simulink model "wlan_phy_rx_pmd"  (Simulink 7.8)
settings: Solver=VariableStepDiscrete  SolverMode=SingleTasking  StartTime=0.0  StopTime=simtime  RelTol=1e-3
PostLoadFcn:
  | wlan_phy_rx_init
InitFcn:
  | wlan_phy_rx_init
StartFcn:
  | wlan_phy_rx_init
values from neighbouring files (symbols this model uses):
  barker_seq20 = 0.95 * circshift(barker_seq20, [0 4]) ./ max(abs(barker_seq20))    [wlan_phy_rx_init.m]
  bit_scrambler_lfsr_bytes = bi2de(reshape(repmat(scr, 1, 8), 8, 127)', 'left-msb')    [wlan_phy_rx_init.m]
  deinterleave_ROM = [deinterleave_ROM interleave_64QAM zeros(1, 512-length(interleave_64QAM))]    [wlan_phy_rx_init.m]
  longCorr_coef_i = [3*real(LTS_corr)]    [wlan_phy_rx_init.m]
  longCorr_coef_q = [3*imag(LTS_corr)]    [wlan_phy_rx_init.m]
  MAX_CP_LEN = 32    [wlan_phy_rx_init.m]
  MAX_NCBPS = 288    [wlan_phy_rx_init.m]
  MAX_NUM_BYTES = 4096    [wlan_phy_rx_init.m]
  MAX_NUM_SC = 64    [wlan_phy_rx_init.m]
  MAX_NUM_SYMS = 600    [wlan_phy_rx_init.m]
  n_bits_preFFT_sampBuff = ceil(log2(4*MAX_NUM_SC))    [wlan_phy_rx_init.m]
  NCBPS_16QAM = 192    [wlan_phy_rx_init.m]
  NCBPS_64QAM = 288    [wlan_phy_rx_init.m]
  NCBPS_BPSK = 48    [wlan_phy_rx_init.m]
  NCBPS_QPSK = 96    [wlan_phy_rx_init.m]
  NDBPS_16QAM12 = 96    [wlan_phy_rx_init.m]
  NDBPS_16QAM34 = 144    [wlan_phy_rx_init.m]
  NDBPS_64QAM23 = 192    [wlan_phy_rx_init.m]
  NDBPS_64QAM34 = 216    [wlan_phy_rx_init.m]
  NDBPS_BPSK12 = 24    [wlan_phy_rx_init.m]
  NDBPS_BPSK34 = 36    [wlan_phy_rx_init.m]
  NDBPS_QPSK12 = 48    [wlan_phy_rx_init.m]
  NDBPS_QPSK34 = 72    [wlan_phy_rx_init.m]
  paylod_vec_samp_time = 8    [wlan_phy_rx_init.m]
  PHY_MIN_PKT_LEN = 14    [wlan_phy_rx_init.m]
  scr_ind_rev = zeros(1,128)    [wlan_phy_rx_init.m]
undefined symbols (not defined in the file or in its folder): gcbh, xlBlockLoadCallback, xlGetNormalizedPeriod, xlipgetpartsetting
libraries: xbsIndex_r4 x3119, simulink x1, xbsEDKLib_r4 x1
(part 5 of 19)

== system wlan_phy_rx_pmd/Chan Est & EQ/Chan Est ==
  note: Scale by 2^-1, since est values
      | are summed from 2 training syms
  x_re : Inport
  x_im : Inport  Port=2
  x_ind : Inport  Port=3
  x_valid : Inport  Port=4
  Sym Ind : Inport  Port=5
  Abs : Abs
  Abs1 : Abs
  Chan Est Offload : Subsystem
  Constant1 : xbsIndex_r4/Constant  const=2  gui_display_data_type=Fixed-point  arith_type=Unsigned  n_bits=2  bin_pt=0  preci_type=Single  exp_width=8  frac_width=24  explicit_period=off  period=8  equ=P=C  opselect=C  inp2=PCIN>>17  opr=+  inp1=P  carry=CIN  dbl_ovrd=off  block_type=constant
  Constant2 : xbsIndex_r4/Constant  const=0  gui_display_data_type=Fixed-point  arith_type=Unsigned  n_bits=2  bin_pt=0  preci_type=Single  exp_width=8  frac_width=24  explicit_period=off  period=8  equ=P=C  opselect=C  inp2=PCIN>>17  opr=+  inp1=P  carry=CIN  dbl_ovrd=off  block_type=constant
  Delay{1..4,7} (5 alike) : xbsIndex_r4/Delay  rst=off  infoeditControl=Selection of Reset will increase slice count due to use of real FFs and instead of SRLs  en=off  latency=2  dbl_ovrd=off  reg_retiming=off  block_type=delay
      settings: xl_area=[0, 0, 0, 0, 0, 0, 0]  block_version=VER_STRING_GOES_HERE
  Gateway Out{1..5,8} (6 alike) : xbsIndex_r4/Gateway Out  inherit_from_input=off  hdl_port=off  timing_constraint=None  locs_specified=off  LOCs={}  UseAsDAC=off  DACChannel='1'  block_type=gatewayout
  H Est Outputs : Scope  NumInputPorts=8
      settings: TimeRange=4000  YMin=-0.5~-1~-0.6000000000000001~-0.5~0~0~0~0  YMax=0.5~0.5~0.3999999999999999~1~80~1~3~0.8  SaveName=ScopeData16
  I Estimate Buffer : Subsystem
  Inverter : xbsIndex_r4/Inverter  en=off  latency=0  dbl_ovrd=off  block_type=inv
  Logical1 : xbsIndex_r4/Logical  logical_function=AND  inputs=2  en=off  latency=0  precision=Full  arith_type=Unsigned  n_bits=16  bin_pt=0  align_bp=on  dbl_ovrd=off  block_type=logical
  Logical2 : xbsIndex_r4/Logical  logical_function=AND  inputs=2  en=off  latency=0  precision=Full  arith_type=Unsigned  n_bits=16  bin_pt=0  align_bp=on  dbl_ovrd=off  block_type=logical
  Mult by LTS : Subsystem
  Q Estimate Buffer : Subsystem
  Real-Imag to Complex : RealImagToComplex
  Real-Imag to Complex1 : RealImagToComplex
  Relational1 : xbsIndex_r4/Relational  mode=a<b  en=off  latency=0  dbl_ovrd=off  block_type=relational
  Relational2 : xbsIndex_r4/Relational  mode=a=b  en=off  latency=0  dbl_ovrd=off  block_type=relational
  Scale : xbsIndex_r4/Scale  scale_factor=-1  dbl_ovrd=off  block_type=scale
  Scale1 : xbsIndex_r4/Scale  scale_factor=-1  dbl_ovrd=off  block_type=scale
  To Float : Subsystem
  " x_I" : Outport
  " x_Q" : Outport  Port=2
  H_I : Outport  Port=3
  H_Q : Outport  Port=4
  " valid" : Outport  Port=5
  " x_ind" : Outport  Port=6
  " sym_ind" : Outport  Port=7
  wires:
  Logical2/1 -> I Estimate Buffer/3(Est_WE), Q Estimate Buffer/3(Est_WE)
  Constant2/1 -> Relational2/1
  x_valid/1 -> Logical2/1, Logical1/1
  Sym Ind/1 -> Relational2/2, Relational1/1, Delay2/1
  Constant1/1 -> Relational1/2
  Relational2/1 -> I Estimate Buffer/4(First Sym), Q Estimate Buffer/4(First Sym)
  Relational1/1 -> Inverter/1, Logical2/2
  Delay7/1 -> Gateway Out4/1, To Float/5(x_ind), Chan Est Offload/1(x_ind)
  Delay1/1 -> Gateway Out5/1, To Float/6(x_valid), Chan Est Offload/4(x_valid)
  Logical1/1 -> Delay1/1
  Inverter/1 -> Logical1/2
  I Estimate Buffer/1(h[n]) -> Scale/1
  Q Estimate Buffer/1(h[n]) -> Scale1/1
  Delay2/1 -> To Float/7(sym_ind), Chan Est Offload/5(sym_ind)
  Delay3/1 -> Gateway Out8/1, To Float/1("x_I ")
  Delay4/1 -> Gateway Out1/1, To Float/2("x_Q ")
  Gateway Out8/1 -> H Est Outputs/1, Real-Imag to Complex/1  "x_I"
  Gateway Out1/1 -> H Est Outputs/2, Real-Imag to Complex/2  "x_Q"
  Gateway Out2/1 -> H Est Outputs/3, Real-Imag to Complex1/1  "H_I"
  Gateway Out3/1 -> H Est Outputs/4, Real-Imag to Complex1/2  "H_Q"
  Gateway Out4/1 -> H Est Outputs/5  "SC Ind"
  Gateway Out5/1 -> H Est Outputs/6  "Valid Out"
  x_ind/1 -> Delay7/1, Q Estimate Buffer/2(Est_Addr), I Estimate Buffer/2(Est_Addr), Mult by LTS/3(x_ind)
  x_re/1 -> Delay3/1, Mult by LTS/1(x_re)
  x_im/1 -> Delay4/1, Mult by LTS/2(x_im)
  Mult by LTS/2(H_im) -> Q Estimate Buffer/1(Est_Data)
  Mult by LTS/1(H_re) -> I Estimate Buffer/1(Est_Data)
  Scale/1 -> Gateway Out2/1, To Float/3("H_I "), Chan Est Offload/2(x_I)
  Scale1/1 -> Gateway Out3/1, To Float/4("H_Q "), Chan Est Offload/3(x_Q)
  To Float/1(" x_I") -> " x_I"/1
  To Float/2(" x_Q") -> " x_Q"/1
  To Float/3(H_I) -> H_I/1
  To Float/4(" H_Q") -> H_Q/1
  To Float/5(" x_ind") -> " x_ind"/1
  To Float/6(" x_valid") -> " valid"/1
  To Float/7(" sym_ind") -> " sym_ind"/1
  Abs/1 -> H Est Outputs/8
  Real-Imag to Complex/1 -> Abs/1
  Real-Imag to Complex1/1 -> Abs1/1
  Abs1/1 -> H Est Outputs/7

== system wlan_phy_rx_pmd/Chan Est & EQ/EQ & Pilot Processing ==
  note: Delay here so phase error is ready just in time for
      | post-EQ symbols at Phase Correction CORDIC
  x_I : Inport
  x_Q : Inport  Port=2
  H_I : Inport  Port=3
  H_Q : Inport  Port=4
  x_valid : Inport  Port=5
  x_ind : Inport  Port=6
  Sym ind : Inport  Port=7
  Convert : xbsIndex_r4/Convert  gui_display_data_type=Fixed-point  arith_type="Signed  (2's comp)"  n_bits=16  bin_pt=14  float_type=Single  exp_bits=8  fraction_bits=24  quantization="Round  (unbiased: +/- Inf)"  overflow=Saturate  en=off  latency=1  dbl_ovrd=off  pipeline=off  block_type=convert
  Convert1 : xbsIndex_r4/Convert  gui_display_data_type=Fixed-point  arith_type="Signed  (2's comp)"  n_bits=16  bin_pt=14  float_type=Single  exp_bits=8  fraction_bits=24  quantization="Round  (unbiased: +/- Inf)"  overflow=Saturate  en=off  latency=1  dbl_ovrd=off  pipeline=off  block_type=convert
  Debug Outputs : Subsystem
  Delay{1,3..5,8..9} (6 alike) : xbsIndex_r4/Delay  rst=off  infoeditControl=Selection of Reset will increase slice count due to use of real FFs and instead of SRLs  en=off  latency=62  dbl_ovrd=off  reg_retiming=off  block_type=delay
  Delay12 : xbsIndex_r4/Delay  rst=off  infoeditControl=Selection of Reset will increase slice count due to use of real FFs and instead of SRLs  en=off  latency=23  dbl_ovrd=off  reg_retiming=off  block_type=delay
  Delay14 : xbsIndex_r4/Delay  rst=off  infoeditControl=Selection of Reset will increase slice count due to use of real FFs and instead of SRLs  en=off  latency=23  dbl_ovrd=off  reg_retiming=off  block_type=delay
  Delay15 : xbsIndex_r4/Delay  rst=off  infoeditControl=Selection of Reset will increase slice count due to use of real FFs and instead of SRLs  en=off  latency=24  dbl_ovrd=off  reg_retiming=off  block_type=delay
  Delay17 : xbsIndex_r4/Delay  rst=off  infoeditControl=Selection of Reset will increase slice count due to use of real FFs and instead of SRLs  en=off  latency=23  dbl_ovrd=off  reg_retiming=off  block_type=delay
  Delay18 : xbsIndex_r4/Delay  rst=off  infoeditControl=Selection of Reset will increase slice count due to use of real FFs and instead of SRLs  en=off  latency=24  dbl_ovrd=off  reg_retiming=off  block_type=delay
  Delay2 : xbsIndex_r4/Delay  rst=off  infoeditControl=Selection of Reset will increase slice count due to use of real FFs and instead of SRLs  en=off  latency=8  dbl_ovrd=off  reg_retiming=off  block_type=delay
  Delay6 : xbsIndex_r4/Delay  rst=off  infoeditControl=Selection of Reset will increase slice count due to use of real FFs and instead of SRLs  en=off  latency=8  dbl_ovrd=off  reg_retiming=off  block_type=delay
  Delay7 : xbsIndex_r4/Delay  rst=off  infoeditControl=Selection of Reset will increase slice count due to use of real FFs and instead of SRLs  en=off  latency=8  dbl_ovrd=off  reg_retiming=off  block_type=delay
  Divide : xbsIndex_r4/Divide  flow_control=NonBlocking  axi_optimize_goal=Resources  algorithm_type=High_Radix  infoedit1=Specify output fraction-width for Fixed-point division.  fractional_width=16  latency=21  rate=1  has_a_tlast=off  has_a_tuser=off  has_b_tlast=off  has_b_tuser=off  en=off  has_result_tready=off  result_tlast_behv=Pass_A_TLAST  has_underflow=off  has_overflow=off  has_invalid_op=off  has_divide_by_zero=off  fpo_op_type=mergeddivide  block_type=divide
      settings: infoedit=Blocking: Wait for data on all inputs, allow back-pressure.<br>NonBlocking: operate every cycle in which all inputs are valid, no back-pressure.
  Divide1 : xbsIndex_r4/Divide  flow_control=NonBlocking  axi_optimize_goal=Resources  algorithm_type=High_Radix  infoedit1=Specify output fraction-width for Fixed-point division.  fractional_width=16  latency=21  rate=1  has_a_tlast=off  has_a_tuser=off  has_b_tlast=off  has_b_tuser=off  en=off  has_result_tready=off  result_tlast_behv=Pass_A_TLAST  has_underflow=off  has_overflow=off  has_invalid_op=off  has_divide_by_zero=off  fpo_op_type=mergeddivide  block_type=divide
      settings: infoedit=Blocking: Wait for data on all inputs, allow back-pressure.<br>NonBlocking: operate every cycle in which all inputs are valid, no back-pressure.
  EQ : Scope  NumInputPorts=8
      settings: TimeRange=6000  YMin=-1~-0.5~0~0~0~-2~-1~0  YMax=1~1~1~100~3~2~2~1  SaveName=ScopeData9
  Gateway Out{,1..7} (8 alike) : xbsIndex_r4/Gateway Out  inherit_from_input=off  hdl_port=off  timing_constraint=None  locs_specified=off  LOCs={}  UseAsDAC=off  DACChannel='1'  block_type=gatewayout
  Mag : Subsystem
  Phase Correction : Subsystem
  Phase Err Calc : Subsystem
  Register3 : xbsIndex_r4/Register  init=0  rst=off  en=off  dbl_ovrd=off  block_type=register
  Register4 : xbsIndex_r4/Register  init=0  rst=off  en=off  dbl_ovrd=off  block_type=register
  Register5 : xbsIndex_r4/Register  init=0  rst=off  en=off  dbl_ovrd=off  block_type=register
  a*conj(b) : Subsystem
  " x_I" : Outport
  " x_Q" : Outport  Port=2
  " x_ind" : Outport  Port=3
  " x_valid" : Outport  Port=4
  " sym ind" : Outport  Port=5
  wires:
  x_ind/1 -> Gateway Out5/1, Delay2/1
  Sym ind/1 -> Gateway Out6/1, Delay7/1
  x_I/1 -> a*conj(b)/1(A_I), Gateway Out/1
  x_Q/1 -> a*conj(b)/2(A_Q), Gateway Out1/1
  H_I/1 -> a*conj(b)/3(B_I), Mag/1(I)
  H_Q/1 -> a*conj(b)/4(B_Q), Mag/2(Q)
  x_valid/1 -> Gateway Out4/1, Delay6/1
  Delay1/1 -> Register4/1
  Delay3/1 -> Register3/1
  Phase Err Calc/1(Sym Phase Err) -> Phase Correction/3(Phase)
  Delay7/1 -> Phase Err Calc/5(sym_ind), Delay9/1
  a*conj(b)/1(P_I) -> Phase Err Calc/1(CP_I), Delay1/1
  a*conj(b)/2(P_Q) -> Phase Err Calc/2(CP_Q), Delay3/1
  Delay2/1 -> Phase Err Calc/4(x_ind), Delay5/1
  Mag/1(M) -> Delay8/1
  Delay8/1 -> Register5/1
  Divide/1 -> Convert/1
  Divide1/1 -> Convert1/1
  Convert/1 -> Phase Correction/1(x_I)
  Convert1/1 -> Phase Correction/2(x_Q)
  Register3/1 -> Divide1/1
  Register4/1 -> Divide/1
  Delay4/1 -> Delay12/1
  Delay12/1 -> Phase Correction/4(x_valid)
  Phase Correction/1(" x_I") -> " x_I"/1, Gateway Out7/1, Debug Outputs/1(I)
  Phase Correction/2(" x_Q") -> " x_Q"/1, Gateway Out2/1, Debug Outputs/2(Q)
  Phase Correction/3(" x_valid") -> " x_valid"/1, Gateway Out3/1, Debug Outputs/3(valid)
  Delay5/1 -> Delay14/1
  Delay14/1 -> Delay15/1
  Delay15/1 -> " x_ind"/1, Debug Outputs/4(x_ind)
  Delay9/1 -> Delay17/1
  Delay17/1 -> Delay18/1
  Delay18/1 -> " sym ind"/1
  Delay6/1 -> Phase Err Calc/3(x_valid), Delay4/1
  Gateway Out6/1 -> EQ/5  "Sym Ind"
  Gateway Out5/1 -> EQ/4  "X Ind"
  Gateway Out4/1 -> EQ/3  "X Valid"
  Gateway Out2/1 -> EQ/7  "EQ Q"
  Gateway Out1/1 -> EQ/2  "X_Q"
  Gateway Out/1 -> EQ/1  "X_I"
  Gateway Out7/1 -> EQ/6  "EQ I"
  Gateway Out3/1 -> EQ/8  "EQ Valid"
  Register5/1 -> Divide/2, Divide1/2

== system wlan_phy_rx_pmd/DSSS Rx/Demod ==
  Start : Inport
  I : Inport  Port=2
  Q : Inport  Port=3
  Bit-to-Byte : Subsystem
  DBPSK Demod : Subsystem
  DSSS Rx : Scope  NumInputPorts=7
      settings: TimeRange=80000  YMin=-0.75~-0.3~0~0.95~40~0~0  YMax=0.75~0.1~1~1.05~48~1~250
  De-spread : Subsystem
  Descramble : Subsystem
  Gateway Out{1..7} (7 alike) : xbsIndex_r4/Gateway Out  inherit_from_input=off  hdl_port=off  timing_constraint=None  locs_specified=off  LOCs={}  UseAsDAC=off  DACChannel='1'  block_type=gatewayout
  Header CRC : Subsystem
  Register8 : xbsIndex_r4/Register  init=0  rst=off  en=on  dbl_ovrd=off  block_type=register
  Sync : Subsystem
  Byte : Outport
  Byte Valid : Outport  Port=2
  Byte Ind : Outport  Port=3
  CRC Calc : Outport  Port=4
  wires:
  Q/1 -> De-spread/2(Q), Gateway Out3/1
  Start/1 -> De-spread/3(Sym Start), Gateway Out6/1
  I/1 -> De-spread/1(I), Gateway Out2/1
  De-spread/1(IQ Valid) -> DBPSK Demod/1(IQ Valid)
  De-spread/2(" I") -> DBPSK Demod/2(I)
  De-spread/3(" Q") -> DBPSK Demod/3(Q)
  DBPSK Demod/1(bit) -> Descramble/1(Bit)
  DBPSK Demod/2(bit valid) -> Descramble/2(Bit Valid)
  Descramble/1(" Bit") -> Register8/1, Sync/1(Bit)
  Descramble/2(" Bit Valid") -> Register8/2, Sync/2(Bit Valid)
  Gateway Out2/1 -> DSSS Rx/1
  Gateway Out3/1 -> DSSS Rx/2
  Gateway Out6/1 -> DSSS Rx/3
  Gateway Out7/1 -> DSSS Rx/4
  Gateway Out1/1 -> DSSS Rx/5
  Gateway Out4/1 -> DSSS Rx/6
  Gateway Out5/1 -> DSSS Rx/7
  Sync/1(Payload Bit) -> Bit-to-Byte/1(Bit), Header CRC/1(Bit)
  Sync/2(" Bit Valid") -> Bit-to-Byte/2(Bit Valid), Header CRC/2(Bit Valid)
  Bit-to-Byte/3(Byte Ind) -> Byte Ind/1, Gateway Out1/1
  Bit-to-Byte/1(Byte) -> Byte/1, Gateway Out5/1
  Bit-to-Byte/2(Byte Valid) -> Byte Valid/1, Gateway Out4/1
  Register8/1 -> Gateway Out7/1
  Header CRC/1(CRC Calc) -> CRC Calc/1

== system wlan_phy_rx_pmd/DSSS Rx/Reset Gen ==
  note: Extend the DSSS reset event long enough to flush
      | the DSSS PHY pipeline and to reduce the
      | chance of spurious double-detections
  Reset : Inport
  Constant1 : xbsIndex_r4/Constant  const=127  gui_display_data_type=Fixed-point  arith_type=Unsigned  n_bits=7  bin_pt=0  preci_type=Single  exp_width=8  frac_width=24  explicit_period=off  period=1  equ=P=C  opselect=C  inp2=PCIN>>17  opr=+  inp1=P  carry=CIN  dbl_ovrd=off  block_type=constant
      settings: block_version=VER_STRING_GOES_HERE
  Counter1 : xbsIndex_r4/Counter  cnt_type=Free Running  cnt_to=Inf  operation=Up  start_count=0  cnt_by_val=1  arith_type=Unsigned  n_bits=7  bin_pt=0  load_pin=off  rst=on  en=on  explicit_period=off  period=1  dbl_ovrd=off  use_behavioral_HDL=off  implementation=Fabric  block_type=counter
  Down Sample3 : xbsIndex_r4/Down Sample  sample_ratio=8  sample_phase="Last Value of Frame  (most efficient)"  en=off  latency=1  dbl_ovrd=off  block_type=dsamp
  Inverter2 : xbsIndex_r4/Inverter  en=off  latency=0  dbl_ovrd=off  block_type=inv
  Logical1 : xbsIndex_r4/Logical  logical_function=OR  inputs=2  en=off  latency=0  precision=Full  arith_type=Unsigned  n_bits=8  bin_pt=2  align_bp=on  dbl_ovrd=off  block_type=logical
      settings: xl_area=[1 0 0 1 0 0 0]  block_version=VER_STRING_GOES_HERE
  Posedge : Subsystem
  Relational1 : xbsIndex_r4/Relational  mode=a=b  en=off  latency=0  dbl_ovrd=off  block_type=relational
  S-R Latch : Subsystem
  S-R Latch2 : Subsystem
  negedge : Subsystem
  DSSS Rx Reset : Outport
  wires:
  Posedge/1(Q) -> S-R Latch/1(S), Logical1/1
  S-R Latch/1(Q) -> Down Sample3/1
  Counter1/1 -> Relational1/1
  Constant1/1 -> Relational1/2
  negedge/1(Q) -> S-R Latch2/1(S)
  S-R Latch2/1(Q) -> Counter1/2, Inverter2/1
  Relational1/1 -> S-R Latch2/2(R), S-R Latch/2(R)
  Inverter2/1 -> Logical1/2
  Reset/1 -> Posedge/1(D), negedge/1(D)
  Down Sample3/1 -> DSSS Rx Reset/1
  Logical1/1 -> Counter1/1

== system wlan_phy_rx_pmd/DSSS Rx/S-R Latch1 ==
  S : Inport
  R : Inport  Port=2
  Constant1 : xbsIndex_r4/Constant  const=1  gui_display_data_type=Boolean  arith_type=Boolean  n_bits=1  bin_pt=0  preci_type=Single  exp_width=8  frac_width=24  explicit_period=off  period=1  equ=P=C  opselect=C  inp2=PCIN>>17  opr=+  inp1=P  carry=CIN  dbl_ovrd=off  block_type=constant
      settings: block_version=VER_STRING_GOES_HERE
  Convert1 : xbsIndex_r4/Convert  gui_display_data_type=Boolean  arith_type=Boolean  n_bits=1  bin_pt=0  float_type=Single  exp_bits=8  fraction_bits=24  quantization=Truncate  overflow=Wrap  en=off  latency=0  dbl_ovrd=off  pipeline=off  block_type=convert
  Convert2 : xbsIndex_r4/Convert  gui_display_data_type=Boolean  arith_type=Boolean  n_bits=1  bin_pt=0  float_type=Single  exp_bits=8  fraction_bits=24  quantization=Truncate  overflow=Wrap  en=off  latency=0  dbl_ovrd=off  pipeline=off  block_type=convert
  Register2 : xbsIndex_r4/Register  init=0  rst=on  en=on  dbl_ovrd=off  block_type=register
      settings: xl_area=[1 1 0 0 0 0 0]  block_version=VER_STRING_GOES_HERE
  Q : Outport
  wires:
  S/1 -> Convert2/1
  Register2/1 -> Q/1
  R/1 -> Convert1/1
  Constant1/1 -> Register2/1
  Convert1/1 -> Register2/2
  Convert2/1 -> Register2/3

== system wlan_phy_rx_pmd/DSSS Rx/SIGNAL Dec ==
  note: Convert to bytes, pad for FCS
  Byte : Inport
  Byte Valid : Inport  Port=2
  Byte Ind : Inport  Port=3
  CRC Calc : Inport  Port=4
  AddSub : xbsIndex_r4/AddSub  mode=Addition  use_carryin=off  use_carryout=off  en=off  latency=0  precision=User Defined  arith_type=Unsigned  n_bits=ceil(log2(MAX_NUM_BYTES))  bin_pt=0  quantization=Truncate  overflow=Wrap  dbl_ovrd=off  use_behavioral_HDL=off  hw_selection=Fabric  pipelined=off  block_type=addsub
  Concat : xbsIndex_r4/Concat  num_inputs=2  dbl_ovrd=off  block_type=concat
  Concat1 : xbsIndex_r4/Concat  num_inputs=2  dbl_ovrd=off  block_type=concat
  Constant1 : xbsIndex_r4/Constant  const=2  gui_display_data_type=Fixed-point  arith_type=Unsigned  n_bits=2  bin_pt=0  preci_type=Single  exp_width=8  frac_width=24  explicit_period=off  period=1  equ=P=C  opselect=C  inp2=PCIN>>17  opr=+  inp1=P  carry=CIN  dbl_ovrd=off  block_type=constant
  Constant2 : xbsIndex_r4/Constant  const=0  gui_display_data_type=Fixed-point  arith_type=Unsigned  n_bits=2  bin_pt=0  preci_type=Single  exp_width=8  frac_width=24  explicit_period=off  period=1  equ=P=C  opselect=C  inp2=PCIN>>17  opr=+  inp1=P  carry=CIN  dbl_ovrd=off  block_type=constant
  Constant3 : xbsIndex_r4/Constant  const=10  gui_display_data_type=Fixed-point  arith_type=Unsigned  n_bits=8  bin_pt=0  preci_type=Single  exp_width=8  frac_width=24  explicit_period=off  period=1  equ=P=C  opselect=C  inp2=PCIN>>17  opr=+  inp1=P  carry=CIN  dbl_ovrd=off  block_type=constant
  Constant4 : xbsIndex_r4/Constant  const=3  gui_display_data_type=Fixed-point  arith_type=Unsigned  n_bits=2  bin_pt=0  preci_type=Single  exp_width=8  frac_width=24  explicit_period=off  period=1  equ=P=C  opselect=C  inp2=PCIN>>17  opr=+  inp1=P  carry=CIN  dbl_ovrd=off  block_type=constant
  Constant5 : xbsIndex_r4/Constant  const=8*1500  gui_display_data_type=Fixed-point  arith_type=Unsigned  n_bits=14  bin_pt=0  preci_type=Single  exp_width=8  frac_width=24  explicit_period=off  period=1  equ=P=C  opselect=C  inp2=PCIN>>17  opr=+  inp1=P  carry=CIN  dbl_ovrd=off  block_type=constant
  Constant6 : xbsIndex_r4/Constant  const=4  gui_display_data_type=Fixed-point  arith_type=Unsigned  n_bits=3  bin_pt=0  preci_type=Single  exp_width=8  frac_width=24  explicit_period=off  period=1  equ=P=C  opselect=C  inp2=PCIN>>17  opr=+  inp1=P  carry=CIN  dbl_ovrd=off  block_type=constant
  Constant7 : xbsIndex_r4/Constant  const=5  gui_display_data_type=Fixed-point  arith_type=Unsigned  n_bits=3  bin_pt=0  preci_type=Single  exp_width=8  frac_width=24  explicit_period=off  period=1  equ=P=C  opselect=C  inp2=PCIN>>17  opr=+  inp1=P  carry=CIN  dbl_ovrd=off  block_type=constant
  Constant8 : xbsIndex_r4/Constant  const=82  gui_display_data_type=Fixed-point  arith_type=Unsigned  n_bits=16  bin_pt=0  preci_type=Single  exp_width=8  frac_width=24  explicit_period=on  period=8  equ=P=C  opselect=C  inp2=PCIN>>17  opr=+  inp1=P  carry=CIN  dbl_ovrd=off  block_type=constant
  DOUT{,2,4..5} (4 alike) : xbsIndex_r4/Register  init=0  rst=on  en=on  dbl_ovrd=off  block_type=register
  DOUT3 : xbsIndex_r4/Register  init=4  rst=on  en=on  dbl_ovrd=off  block_type=register
  Delay1 : xbsIndex_r4/Delay  rst=off  infoeditControl=Selection of Reset will increase slice count due to use of real FFs and instead of SRLs  en=off  latency=1  dbl_ovrd=off  reg_retiming=off  block_type=delay
  Display : Display
  Display1 : Display
  Down Sample2 : xbsIndex_r4/Down Sample  sample_ratio=8  sample_phase="Last Value of Frame  (most efficient)"  en=off  latency=1  dbl_ovrd=off  block_type=dsamp
  Gateway Out : xbsIndex_r4/Gateway Out  inherit_from_input=off  hdl_port=off  timing_constraint=None  locs_specified=off  LOCs={}  UseAsDAC=off  DACChannel='1'  block_type=gatewayout
  Gateway Out1 : xbsIndex_r4/Gateway Out  inherit_from_input=off  hdl_port=off  timing_constraint=None  locs_specified=off  LOCs={}  UseAsDAC=off  DACChannel='1'  block_type=gatewayout
  Inverter1 : xbsIndex_r4/Inverter  en=off  latency=0  dbl_ovrd=off  block_type=inv
  Logical{,1..3,7..10} (8 alike) : xbsIndex_r4/Logical  logical_function=AND  inputs=2  en=off  latency=0  precision=Full  arith_type=Unsigned  n_bits=16  bin_pt=0  align_bp=on  dbl_ovrd=off  block_type=logical
  Logical6 : xbsIndex_r4/Logical  logical_function=OR  inputs=3  en=off  latency=0  precision=Full  arith_type=Unsigned  n_bits=16  bin_pt=0  align_bp=on  dbl_ovrd=off  block_type=logical
  Relational{1..2,4,6..7} (5 alike) : xbsIndex_r4/Relational  mode=a=b  en=off  latency=0  dbl_ovrd=off  block_type=relational
  Relational3 : xbsIndex_r4/Relational  mode=a!=b  en=off  latency=0  dbl_ovrd=off  block_type=relational
  Relational5 : xbsIndex_r4/Relational  mode=a>b  en=off  latency=0  dbl_ovrd=off  block_type=relational
  Relational8 : xbsIndex_r4/Relational  mode=a!=b  en=off  latency=0  dbl_ovrd=off  block_type=relational
  Shift : xbsIndex_r4/Shift  shift_dir=Right  shift_bits=3  en=off  latency=0  precision=Full  arith_type="Signed  (2's comp)"  n_bits=16  bin_pt=14  quantization=Truncate  overflow=Wrap  dbl_ovrd=off  block_type=shift
  LENGTH : Outport
  Valid Header : Outport  Port=2
  Error : Outport  Port=3
  wires:
  Constant2/1 -> Relational2/2
  Relational1/1 -> Logical/2
  Relational2/1 -> Logical2/2
  Logical/1 -> DOUT2/3
  Logical2/1 -> DOUT/3
  Byte/1 -> DOUT/1, DOUT2/1, DOUT3/1, DOUT4/1, DOUT5/1
  [DSSS_RX_RESET] -> DOUT/2, DOUT2/2, DOUT3/2, DOUT4/2, DOUT5/2
  Byte Valid/1 -> Logical2/1, Logical/1, Logical3/1, Logical8/1, Logical7/1
  Constant1/1 -> Relational1/2
  Byte Ind/1 -> Relational1/1, Relational4/1, Relational7/1, Relational6/1, Relational2/1
  DOUT/1 -> Relational3/1, Gateway Out1/1
  Constant3/1 -> Relational3/2
  Relational4/1 -> Logical3/2
  Constant4/1 -> Relational4/2
  Logical3/1 -> DOUT3/3
  DOUT2/1 -> Concat/2
  DOUT3/1 -> Concat/1
  Concat/1 -> Gateway Out/1, Relational5/1, Shift/1
  Constant5/1 -> Relational5/2
  Relational5/1 -> Logical6/2
  Relational3/1 -> Logical6/1
  Gateway Out/1 -> Display/1
  Gateway Out1/1 -> Display1/1
  Constant6/1 -> Relational6/2
  Logical8/1 -> DOUT4/3, Delay1/1
  Relational6/1 -> Logical7/2
  Relational7/1 -> Logical8/2
  Constant7/1 -> Relational7/2
  DOUT4/1 -> Concat1/1
  DOUT5/1 -> Concat1/2
  Concat1/1 -> Relational8/1
  CRC Calc/1 -> Relational8/2
  Relational8/1 -> Logical9/1
  Delay1/1 -> Logical9/2, Logical10/2, Logical1/2
  Logical9/1 -> Logical6/3
  Logical7/1 -> DOUT5/3
  [regRx_DSSS_LENGTH_PAD] -> Down Sample2/1
  Down Sample2/1 -> AddSub/2
  AddSub/1 -> LENGTH/1
  Logical6/1 -> Inverter1/1, Logical1/1
  Inverter1/1 -> Logical10/1
  Logical10/1 -> Valid Header/1
  Logical1/1 -> Error/1
  Shift/1 -> AddSub/1

== system wlan_phy_rx_pmd/DSSS Rx/Sym Sync ==
  En : Inport
  Rx I : Inport  Port=2
  Rx Q : Inport  Port=3
  ASR : xbsIndex_r4/Addressable Shift Register  infer_latency=on  depth=2  initVector=[0]  en=off  dbl_ovrd=off  use_behavioral_HDL=off  Optimization=Resource  block_type=addrsr
      settings: infoedit="Delay of configurable length.  Any element in the delay line can be addressed and driven onto the output port.<br><br>Hardware notes: This block is implemented using SRL16s. If Virtex-4 or Spartan-3 device is used, multiple SRLC16s are cascaded together."
  Abs : Subsystem
  Convert : xbsIndex_r4/Convert  gui_display_data_type=Fixed-point  arith_type="Signed  (2's comp)"  n_bits=20  bin_pt=14  float_type=Single  exp_bits=8  fraction_bits=24  quantization=Truncate  overflow=Wrap  en=off  latency=0  dbl_ovrd=off  pipeline=off  block_type=convert
  DSSS Corr : Scope  NumInputPorts=7
      settings: TimeRange=80000  YMin=-0.25~-1.5~0~0~0~0~0  YMax=0.15~4.5~20~1~1~1~1
  Delay : xbsIndex_r4/Delay  rst=off  infoeditControl=Selection of Reset will increase slice count due to use of real FFs and instead of SRLs  en=off  latency=20  dbl_ovrd=off  reg_retiming=off  block_type=delay
  Delay1 : xbsIndex_r4/Delay  rst=off  infoeditControl=Selection of Reset will increase slice count due to use of real FFs and instead of SRLs  en=off  latency=20  dbl_ovrd=off  reg_retiming=off  block_type=delay
  Display : Display
  Down Sample1 : xbsIndex_r4/Down Sample  sample_ratio=8  sample_phase="Last Value of Frame  (most efficient)"  en=off  latency=1  dbl_ovrd=off  block_type=dsamp
  Down Sample2 : xbsIndex_r4/Down Sample  sample_ratio=8  sample_phase="Last Value of Frame  (most efficient)"  en=off  latency=1  dbl_ovrd=off  block_type=dsamp
  "FIR Compiler 6.3 " : xbsIndex_r4/FIR Compiler 6.3   coefficientvector=fliplr(barker_seq20)  coefficient_sets=1  coefficient_reload=off  filter_type=Single_Rate  rate_change_type=Integer  interpolation_rate=1  decimation_rate=1  zero_pack_factor=1  channel_sequence=Basic  number_channels=1  pattern_list='P4-0,P4-1,P4-2,P4-3,P4-4'  number_paths=2  ratespecification=Maximum_Possible  sampleperiod=8  hardwareoversamplingrate=1  coefficient_sign=Signed  quantization=Maximize_Dynamic_Range  coefficient_width=16  bestprecision=on  coefficient_fractional_bits=15  coefficient_structure=Inferred  output_rounding_mode=Truncate_LSBs  output_width=16  filter_architecture=Systolic_Multiply_Accumulate  optimization_goal=Area  data_buffer_type=Automatic  coefficient_buffer_type=Automatic  input_buffer_type=Automatic  output_buffer_type=Automatic  preference_for_other_storage=Automatic  multi_column_support=Automatic  columnconfig='20'  inter_column_pipe_length=4  data_has_tlast=Not_Required  m_data_has_tready=off  s_data_has_fifo=off  s_data_has_tuser=Not_Required  m_data_has_tuser=Not_Required  s_config_sync_mode=On_Vector  s_config_method=Single  num_reload_slots=1  has_aclken=off  has_aresetn=off  infoeditControl=ARESETn must be asserted for a minimum of 2 cycles  trim_axipin_name=on  passband_min=0.0  passband_max=0.5  stopband_min=0.5  stopband_max=1.0  filter_selection=1  gui_behaviour=Sysgen_GUI  data_sign=Signed  data_width=16  data_fractional_bits=0  data_tuser_width=1  wrapper_available=true  ip_wrap_arbitrary_binary_point=true  ip_name=FIR Compiler  ip_version=6.3  dsptool_ready=true  ipcore_usecache=true  ipcore_useipmodelcache=true  ipcore_xco_need_fpga_part=true  port_translation_map={ 'aclken' => 'en', 'aresetn' => 'rst' }  run_core_at_system_period=true  ipcore_fpga_part=xlipgetpartsetting(gcb, {'virtex6', 'xc6vlx75t', '-3', 'ff784'})  block_type=fir_compiler_v6_3
      settings: infoedit=Maximum Possible : Maximizes oversampling rate. Abstracted input handshake (no s_data_tvalid) and automatic rate propagation.<br>Hardware Oversampling Rate : Specifies oversampling rate relative to the input sample period. Abstracted input handshake (no s_data_tvalid) and automatic rate propagation.<br>Sample Period : Specifies absolute oversampling rate. No input handshake abstraction (exposes s_data_tvalid) and no rate propagation.
  Gateway Out{,1..7} (8 alike) : xbsIndex_r4/Gateway Out  inherit_from_input=off  hdl_port=off  timing_constraint=None  locs_specified=off  LOCs={}  UseAsDAC=off  DACChannel='1'  block_type=gatewayout
  Logical : xbsIndex_r4/Logical  logical_function=AND  inputs=4  en=off  latency=0  precision=Full  arith_type=Unsigned  n_bits=16  bin_pt=0  align_bp=on  dbl_ovrd=off  block_type=logical
  Relational : xbsIndex_r4/Relational  mode=a<b  en=off  latency=0  dbl_ovrd=off  block_type=relational
  Sym : Outport
  tag scope: CS_DSSS_Corr=global
  wires:
  Rx Q/1 -> Gateway Out/1, "FIR Compiler 6.3 "/2
  Gateway Out/1 -> DSSS Corr/1
  "FIR Compiler 6.3 "/2 -> Abs/1(Valid)
  "FIR Compiler 6.3 "/3 -> Abs/2(I), Gateway Out1/1
  "FIR Compiler 6.3 "/4 -> Abs/3(Q)
  Gateway Out1/1 -> DSSS Corr/2
  Abs/1(A) -> Relational/2, Gateway Out2/1, Convert/1
  [regRx_DSSS_RX_CODE_CORR_THRESH] -> Down Sample2/1
  Rx I/1 -> "FIR Compiler 6.3 "/1
  Relational/1 -> ASR/1
  Gateway Out2/1 -> DSSS Corr/3
  Gateway Out3/1 -> Display/1
  ASR/1 -> Logical/1, Delay/1, Gateway Out4/1
  [regRx_DSSS_RX_DESPREAD_DLY] -> Down Sample1/1
  Delay/1 -> Delay1/1, Logical/2, Gateway Out5/1
  Delay1/1 -> Logical/3, Gateway Out6/1
  En/1 -> Logical/4
  Gateway Out4/1 -> DSSS Corr/4
  Gateway Out5/1 -> DSSS Corr/5
  Gateway Out6/1 -> DSSS Corr/6
  Gateway Out7/1 -> DSSS Corr/7
  Logical/1 -> Sym/1, Gateway Out7/1
  Down Sample1/1 -> ASR/2
  Down Sample2/1 -> Relational/1, Gateway Out3/1
  Convert/1 -> [CS_DSSS_Corr]

== system wlan_phy_rx_pmd/Detect & Decode/Ctrl ==
  Reset : Inport
  OFDM Sym Ind : Inport  Port=2
  Constant1 : xbsIndex_r4/Constant  const=1  gui_display_data_type=Fixed-point  arith_type=Unsigned  n_bits=2  bin_pt=0  preci_type=Single  exp_width=8  frac_width=24  explicit_period=off  period=1  equ=P=C  opselect=C  inp2=PCIN>>17  opr=+  inp1=P  carry=CIN  dbl_ovrd=off  block_type=constant
  Constant3 : xbsIndex_r4/Constant  const=2  gui_display_data_type=Fixed-point  arith_type=Unsigned  n_bits=2  bin_pt=0  preci_type=Single  exp_width=8  frac_width=24  explicit_period=off  period=1  equ=P=C  opselect=C  inp2=PCIN>>17  opr=+  inp1=P  carry=CIN  dbl_ovrd=off  block_type=constant
  Logical1 : xbsIndex_r4/Logical  logical_function=OR  inputs=2  en=off  latency=0  precision=Full  arith_type=Unsigned  n_bits=8  bin_pt=2  align_bp=on  dbl_ovrd=off  block_type=logical
      settings: xl_area=[1 0 0 1 0 0 0]  block_version=VER_STRING_GOES_HERE
  Posedge : Subsystem
  Posedge1 : Subsystem
  Relational1 : xbsIndex_r4/Relational  mode=a>b  en=off  latency=0  dbl_ovrd=off  block_type=relational
  Relational2 : xbsIndex_r4/Relational  mode=a>b  en=off  latency=0  dbl_ovrd=off  block_type=relational
  Reset Demod : Outport
  Start Decoder : Outport  Port=2
  tag scope: CS_DEMOD_CTRL_RESET=global, CS_DEMOD_CTRL_RESET_DEMOD=global
  wires:
  Constant1/1 -> Relational2/2
  Relational2/1 -> Posedge1/1(D)
  Constant3/1 -> Relational1/2
  Relational1/1 -> Posedge/1(D)
  OFDM Sym Ind/1 -> Relational1/1, Relational2/1
  Posedge1/1(Q) -> Logical1/2
  Posedge/1(Q) -> Start Decoder/1
  Logical1/1 -> Reset Demod/1, [CS_DEMOD_CTRL_RESET_DEMOD]
  Reset/1 -> Logical1/1, [CS_DEMOD_CTRL_RESET]

== system wlan_phy_rx_pmd/Detect & Decode/De-Interleave & De-Puncture ==
  note: This RAM contains one OFDM symbol's worth of coded bits, represented as log-likelihood ratios (LLR).
      | These soft bits are written to the RAM in pairs (except for BPSK) in linear order. Once a full symbol's
      | worth of bits is written the same bits are then read in de-interleaved order. The bits are read in pairs and
      | fed directly into the rate 1/2 Viterbi decoder. Operating on pairs-of-bits here significantly decreases the latency
      | of the de-interleaving process and is necessary to finish decoding 64QAM 3/4 payloads inside the
      | alloted 6usec post-Rx time.
  LLR A : Inport
  LLR B : Inport  Port=2
  tvalid : Inport  Port=3
  mod_sel : Inport  Port=4
  Reset : Inport  Port=5
  rate_sel : Inport  Port=6
  DeInt : Scope  NumInputPorts=9
      settings: TimeRange=70.99443917115605  YMin=-1~0.95~-1~-1~-1~45.6~-1~-4.2~-1  YMax=1~1.05~1~1~1~50.4~1~-3.8~1  SaveName=ScopeData7
  Delay3 : xbsIndex_r4/Delay  rst=off  infoeditControl=Selection of Reset will increase slice count due to use of real FFs and instead of SRLs  en=off  latency=1  dbl_ovrd=off  reg_retiming=off  block_type=delay
  Gateway Out{1..9} (9 alike) : xbsIndex_r4/Gateway Out  inherit_from_input=off  hdl_port=off  timing_constraint=None  locs_specified=off  LOCs={}  UseAsDAC=off  DACChannel='1'  block_type=gatewayout
  Inverter1 : xbsIndex_r4/Inverter  en=off  latency=0  dbl_ovrd=off  block_type=inv
      settings: xl_area=[0 0 0 0 0 0 0]  block_version=VER_STRING_GOES_HERE
  LLR-per-bit RAM : xbsIndex_r4/Dual Port RAM  depth=2^(ceil(log2(MAX_NCBPS)))  initVector=0  distributed_mem=Block RAM  init_a=0  init_b=0  rst_a=on  rst_b=on  en_a=off  en_b=off  latency=1  write_mode_A=Read After Write  write_mode_B=Read After Write  dbl_ovrd=off  optimize=Area  block_type=dpram
  Logical3 : xbsIndex_r4/Logical  logical_function=OR  inputs=2  en=off  latency=0  precision=Full  arith_type=Unsigned  n_bits=16  bin_pt=0  align_bp=on  dbl_ovrd=off  block_type=logical
  Logical4 : xbsIndex_r4/Logical  logical_function=OR  inputs=2  en=off  latency=0  precision=Full  arith_type=Unsigned  n_bits=16  bin_pt=0  align_bp=on  dbl_ovrd=off  block_type=logical
  Mux1 : xbsIndex_r4/Mux  inputs=2  en=off  latency=0  precision=Full  arith_type=Unsigned  n_bits=16  bin_pt=14  quantization=Truncate  overflow=Wrap  dbl_ovrd=off  block_type=mux
  Mux2 : xbsIndex_r4/Mux  inputs=2  en=off  latency=0  precision=Full  arith_type=Unsigned  n_bits=16  bin_pt=14  quantization=Truncate  overflow=Wrap  dbl_ovrd=off  block_type=mux
  Rd Addr Gen : Subsystem
  Wr Addr Gen : Subsystem
  " LLR A" : Outport
  " LLR B" : Outport  Port=2
  " tvalid" : Outport  Port=3
  tag scope: CS_DeInt_ModSel=global, CS_DeInt_RdAddr=global, CS_DeInt_Reset=global, CS_DeInt_Stuff0=global, CS_DeInt_Vin=global, CS_DeInt_Vout=global, CS_DeInt_WrAddr=global, CS_DeInt_WrEn=global, CS_Deint_Addr_A=global, CS_Deint_Wen=global
  wires:
  tvalid/1 -> Wr Addr Gen/1(tvalid), Gateway Out5/1  "tvalid in"
  mod_sel/1 -> Wr Addr Gen/2(mod_sel), Rd Addr Gen/2(mod_sel), Gateway Out1/1  "mod_sel"
  Reset/1 -> Wr Addr Gen/3(Reset), Rd Addr Gen/3(Reset), Gateway Out9/1
  Wr Addr Gen/4(Block Wr Done) -> Rd Addr Gen/1(Block Wr Done), Gateway Out4/1
  Gateway Out5/1 -> DeInt/1  "tvalid in"
  Gateway Out1/1 -> DeInt/2  "mod_sel"
  Gateway Out2/1 -> DeInt/5  "Addr A"
  Gateway Out3/1 -> DeInt/3  "Wr En"
  Gateway Out4/1 -> DeInt/4  "Block Wr Done"
  Gateway Out6/1 -> DeInt/6  "Addr B"
  Gateway Out7/1 -> DeInt/7  "tvalid out"
  Gateway Out8/1 -> DeInt/8  "Mem out"
  rate_sel/1 -> Rd Addr Gen/4(rate_sel)
  Gateway Out9/1 -> DeInt/9  "Reset In"
  LLR-per-bit RAM/2 -> " LLR B"/1
  Mux2/1 -> Gateway Out2/1, LLR-per-bit RAM/1, [CS_Deint_Addr_A]
  Mux1/1 -> LLR-per-bit RAM/4, Gateway Out6/1
  Wr Addr Gen/3(Wr En) -> Mux1/1, Mux2/1, LLR-per-bit RAM/6, LLR-per-bit RAM/3, [CS_Deint_Wen], Gateway Out3/1
  Wr Addr Gen/1(Wr Addr A) -> Mux2/3
  Wr Addr Gen/2(Wr Addr B) -> Mux1/3
  LLR A/1 -> LLR-per-bit RAM/2
  LLR B/1 -> LLR-per-bit RAM/5
  LLR-per-bit RAM/1 -> Gateway Out8/1, " LLR A"/1
  Rd Addr Gen/1(Rd Addr A) -> Mux2/2
  Rd Addr Gen/2(Rd Addr B) -> Mux1/2
  Rd Addr Gen/5(Vout) -> Delay3/1, Inverter1/1
  Delay3/1 -> " tvalid"/1, Gateway Out7/1
  Rd Addr Gen/3(Skip A) -> Logical3/1
  Rd Addr Gen/4(Skip B) -> Logical4/1
  Logical3/1 -> LLR-per-bit RAM/7
  Logical4/1 -> LLR-per-bit RAM/8
  Inverter1/1 -> Logical4/2, Logical3/2

== system wlan_phy_rx_pmd/Detect & Decode/Decode ==
  note: Required to avoid combinational loop
      | back to BB reset input
  Reset : Inport
  LLR A : Inport  Port=2
  LLR B : Inport  Port=3
  Vin : Inport  Port=4
  Start : Inport  Port=5
  Assert11 : xbsIndex_r4/Assert  assert_type=off  type_source=Explicitly  gui_display_data_type=Fixed-point  arith_type="Signed  (2's comp)"  n_bits=16  bin_pt=14  preci_type=Single  exp_width=8  frac_width=24  assert_rate=on  rate_source=Explicitly  period=1  output_port=on  block_type=assert
      settings: block_version=VER_STRING_GOES_HERE
  Byte Count : Subsystem
  Convert3 : xbsIndex_r4/Convert  gui_display_data_type=Boolean  arith_type=Boolean  n_bits=1  bin_pt=0  float_type=Single  exp_bits=8  fraction_bits=24  quantization=Truncate  overflow=Wrap  en=off  latency=0  dbl_ovrd=off  pipeline=off  block_type=convert
  Ctrl : Subsystem
  Decoder I/O : Scope  NumInputPorts=12
      settings: TimeRange=9204  YMin=-7.5~-7.5~0~0~0~0~0~0~0~-5~-5~-5  YMax=7.5~7.5~1~1~1~200~1~1~200~5~5~5  SaveName=ScopeData3
  Delay : xbsIndex_r4/Delay  rst=off  infoeditControl=Selection of Reset will increase slice count due to use of real FFs and instead of SRLs  en=off  latency=1  dbl_ovrd=off  reg_retiming=off  block_type=delay
  Gateway Out{1..6,8..12} (11 alike) : xbsIndex_r4/Gateway Out  inherit_from_input=off  hdl_port=off  timing_constraint=None  locs_specified=off  LOCs={}  UseAsDAC=off  DACChannel='1'  block_type=gatewayout
  Logical1 : xbsIndex_r4/Logical  logical_function=OR  inputs=3  en=off  latency=0  precision=Full  arith_type=Unsigned  n_bits=16  bin_pt=0  align_bp=on  dbl_ovrd=off  block_type=logical
  Logical2 : xbsIndex_r4/Logical  logical_function=OR  inputs=2  en=off  latency=0  precision=Full  arith_type=Unsigned  n_bits=16  bin_pt=0  align_bp=on  dbl_ovrd=off  block_type=logical
  Register : xbsIndex_r4/Register  init=0  rst=off  en=off  dbl_ovrd=off  block_type=register
  Register4 : xbsIndex_r4/Register  init=0  rst=off  en=off  dbl_ovrd=off  block_type=register
  Register5 : xbsIndex_r4/Register  init=0  rst=off  en=off  dbl_ovrd=off  block_type=register
  Reinterpret : xbsIndex_r4/Reinterpret  force_arith_type=on  arith_type=Unsigned  force_bin_pt=on  bin_pt=0  block_type=reinterpret
  Reinterpret2 : xbsIndex_r4/Reinterpret  force_arith_type=on  arith_type=Unsigned  force_bin_pt=on  bin_pt=0  block_type=reinterpret
  "SIGNAL decode " : Subsystem
  Safe ToUint8 : Subsystem
  Stretch : Subsystem
  Viberbi Decoder : xbsIndex_r4/Black Box  init_code=vb_decoder_top_config  sim_method=ISE Simulator  verbose=off  block_type=blackbox2
  Byte : Outport
  Byte Valid : Outport  Port=2
  Byte Index : Outport  Port=3
  Last Byte : Outport  Port=4
  " Reset" : Outport  Port=5
  Mod Order : Outport  Port=6
  Code Rate : Outport  Port=7
  tag scope: CS_DEC_BYTE_INDEX=global, CS_DEC_BYTE_OUT=global, CS_DEC_BYTE_VALID_OUT=global, CS_DEC_END=global, CS_DEC_LLR_b0=global, CS_DEC_LLR_b1=global, CS_DEC_RESET=global, CS_DEC_START=global, CS_DEC_VIN=global, CS_DEC_VOUT=global
  wires:
  Register/1 -> Reinterpret/1, Gateway Out11/1, [CS_DEC_LLR_b1]
  Register4/1 -> Reinterpret2/1, Gateway Out10/1, [CS_DEC_LLR_b0]
  Register5/1 -> Ctrl/1(dec_vin)
  Viberbi Decoder/2 -> Convert3/1, Ctrl/5(dec_vout)
  Viberbi Decoder/3 -> Safe ToUint8/1(A)
  Start/1 -> Logical2/1, Byte Count/2(Decode Start), Ctrl/3(Start)
  Safe ToUint8/1(B) -> "SIGNAL decode "/3(data_byte), Byte/1, [CS_DEC_BYTE_OUT]
  Byte Count/2(Byte Index) -> "SIGNAL decode "/1(byte_ind), Byte Index/1, [CS_DEC_BYTE_INDEX], Gateway Out5/1
  Gateway Out1/1 -> Decoder I/O/10  "Vout"
  Gateway Out5/1 -> Decoder I/O/9  "Byte Index"
  Gateway Out6/1 -> Decoder I/O/8  "Last Byte"
  Gateway Out4/1 -> Decoder I/O/5  "LLR Valid"
  Gateway Out2/1 -> Decoder I/O/6  "Start"
  Gateway Out3/1 -> Decoder I/O/7  "End"
  "SIGNAL decode "/3(code_rate) -> Code Rate/1
  "SIGNAL decode "/4(mod_order) -> Mod Order/1
  Assert11/1 -> Logical2/2, Stretch/1(D)
  "SIGNAL decode "/2(SIGNAL err) -> Logical1/2
  Logical1/1 -> Assert11/1
  Gateway Out8/1 -> Decoder I/O/11  "Reset Out"
  Logical2/1 -> "SIGNAL decode "/4(rst)
  Vin/1 -> Register5/1
  LLR B/1 -> Register4/1
  LLR A/1 -> Register/1
  Byte Count/3(Last Byte) -> Last Byte/1, Delay/1, Gateway Out6/1
  "SIGNAL decode "/1(last_byte_ind) -> Byte Count/3(Last Byte Ind), Ctrl/4(Num Bytes)
  Byte Count/1(" Byte Valid") -> Byte Valid/1, [CS_DEC_BYTE_VALID_OUT], Gateway Out1/1, "SIGNAL decode "/2(data_valid)
  Stretch/1(Q) -> " Reset"/1, Gateway Out8/1
  Convert3/1 -> Byte Count/1(Byte Valid), [CS_DEC_VOUT]
  Gateway Out9/1 -> Decoder I/O/4  "Reset#"
  [RX_GLOBAL_RESET] -> Logical1/3
  Delay/1 -> Logical1/1
  Gateway Out10/1 -> Decoder I/O/1  "LLR B0"
  Gateway Out11/1 -> Decoder I/O/2  "LLR B1"
  Gateway Out12/1 -> Decoder I/O/3  "Early Trace"
  Ctrl/1(vin) -> Viberbi Decoder/3, Gateway Out4/1, [CS_DEC_VIN]
  Ctrl/2(rst#) -> Viberbi Decoder/4, Gateway Out9/1, [CS_DEC_RESET]
  Ctrl/3(start) -> Viberbi Decoder/5, Gateway Out2/1, [CS_DEC_START]
  Ctrl/4(pkt_end) -> Viberbi Decoder/6, Gateway Out3/1, [CS_DEC_END]
  Ctrl/5(early_trace_en) -> Viberbi Decoder/7, Gateway Out12/1
  Reset/1 -> Ctrl/2(Reset)
  Reinterpret/1 -> Viberbi Decoder/1
  Reinterpret2/1 -> Viberbi Decoder/2

== system wlan_phy_rx_pmd/Detect & Decode/Descramble ==
  Byte : Inport
  Byte Valid : Inport  Port=2
  Byte Index : Inport  Port=3
  Last Byte : Inport  Port=4
  DOUT : xbsIndex_r4/Register  init=0  rst=off  en=on  dbl_ovrd=off  block_type=register
  Delay{,1,3..4} (4 alike) : xbsIndex_r4/Delay  rst=off  infoeditControl=Selection of Reset will increase slice count due to use of real FFs and instead of SRLs  en=off  latency=3  dbl_ovrd=off  reg_retiming=off  block_type=delay
  Descram : Scope  NumInputPorts=6
      settings: TimeRange=10000  YMin=0~0~32.5~-1~-1~0  YMax=1~275~52.5~1~1~250  SaveName=ScopeData18
  Descram Byte Calc : Subsystem
  Endian Swap : Subsystem
  Gateway Out{1..9} (9 alike) : xbsIndex_r4/Gateway Out  inherit_from_input=off  hdl_port=off  timing_constraint=None  locs_specified=off  LOCs={}  UseAsDAC=off  DACChannel='1'  block_type=gatewayout
  Logical1 : xbsIndex_r4/Logical  logical_function=XOR  inputs=2  en=off  latency=0  precision=Full  arith_type=Unsigned  n_bits=16  bin_pt=0  align_bp=on  dbl_ovrd=off  block_type=logical
  To Workspace : ToWorkspace  VariableName=byteout  SampleTime=-1  SaveFormat=Array
  To Workspace1 : ToWorkspace  VariableName=byteout_valid  SampleTime=-1  SaveFormat=Array
  To Workspace2 : ToWorkspace  VariableName=byteout_index  SampleTime=-1  SaveFormat=Array
  " Byte" : Outport
  " Byte Valid" : Outport  Port=2
  " Byte Index" : Outport  Port=3
  " Last Byte" : Outport  Port=4
  wires:
  Gateway Out4/1 -> Descram/6
  DOUT/1 -> Gateway Out4/1
  Delay/1 -> Logical1/1, Gateway Out9/1
  Gateway Out1/1 -> Descram/3
  Gateway Out6/1 -> Descram/2
  Gateway Out5/1 -> Descram/1
  Delay4/1 -> Gateway Out1/1, " Byte Index"/1, Gateway Out7/1
  Delay3/1 -> Gateway Out5/1, " Byte Valid"/1, DOUT/2, Gateway Out3/1
  Logical1/1 -> Endian Swap/1(B)
  Descram Byte Calc/1(Descram Val) -> Logical1/2, Gateway Out8/1
  Byte/1 -> Descram Byte Calc/1(data_byte), Delay/1
  Byte Index/1 -> Descram Byte Calc/3(data_ind), Delay4/1
  Byte Valid/1 -> Descram Byte Calc/2(data_valid), Delay3/1
  Endian Swap/1(I) -> " Byte"/1, Gateway Out6/1, DOUT/1, Gateway Out2/1
  Last Byte/1 -> Delay1/1
  Delay1/1 -> " Last Byte"/1
  Gateway Out3/1 -> To Workspace1/1
  Gateway Out2/1 -> To Workspace/1
  Gateway Out7/1 -> To Workspace2/1
  Gateway Out8/1 -> Descram/4
  Gateway Out9/1 -> Descram/5

== system wlan_phy_rx_pmd/Detect & Decode/Mod Rate Sel ==
  OFDM Sym Ind : Inport
  Payload Mod : Inport  Port=2
  Constant4 : xbsIndex_r4/Constant  const=0  gui_display_data_type=Fixed-point  arith_type=Unsigned  n_bits=2  bin_pt=0  preci_type=Single  exp_width=8  frac_width=24  explicit_period=on  period=1  equ=P=C  opselect=C  inp2=PCIN>>17  opr=+  inp1=P  carry=CIN  dbl_ovrd=off  block_type=constant
  Constant5 : xbsIndex_r4/Constant  const=3  gui_display_data_type=Fixed-point  arith_type=Unsigned  n_bits=2  bin_pt=0  preci_type=Single  exp_width=8  frac_width=24  explicit_period=off  period=1  equ=P=C  opselect=C  inp2=PCIN>>17  opr=+  inp1=P  carry=CIN  dbl_ovrd=off  block_type=constant
  Mux3 : xbsIndex_r4/Mux  inputs=2  en=off  latency=0  precision=Full  arith_type=Unsigned  n_bits=16  bin_pt=14  quantization=Truncate  overflow=Wrap  dbl_ovrd=off  block_type=mux
  Relational3 : xbsIndex_r4/Relational  mode=a>=b  en=off  latency=0  dbl_ovrd=off  block_type=relational
  Mod Sel : Outport
  wires:
  Constant5/1 -> Relational3/2
  Relational3/1 -> Mux3/1
  Constant4/1 -> Mux3/2
  OFDM Sym Ind/1 -> Relational3/1
  Mux3/1 -> Mod Sel/1
  Payload Mod/1 -> Mux3/3
